simulink model "gate_V31_2016a"  (R2016a) (continued)
== system gate_V31_2016a/Gate/HardwareInputWrapper/MIL_Gate_A == (continued)
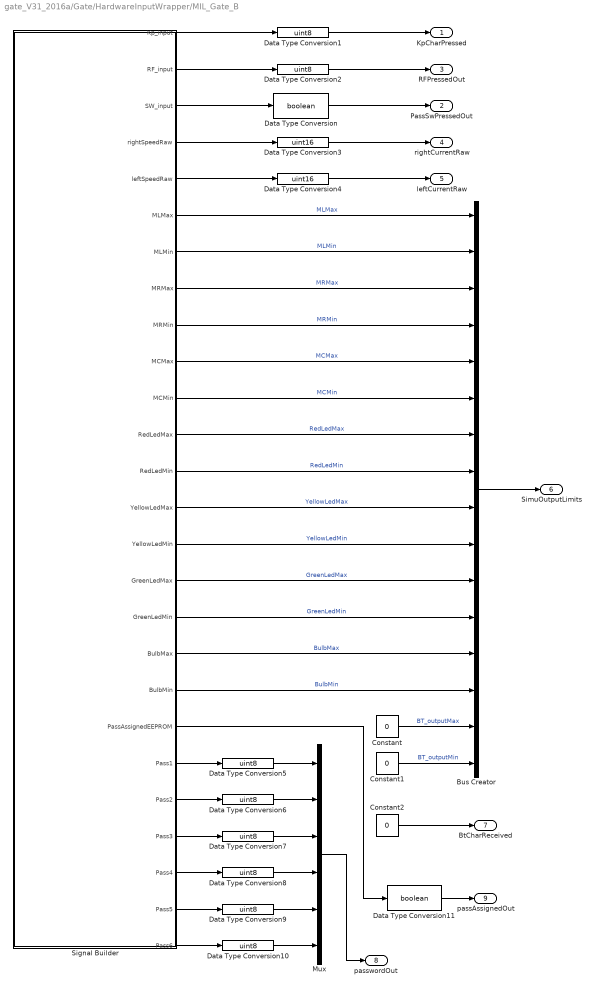
== system gate_V31_2016a/Gate/HardwareInputWrapper/MIL_Gate_B ==
  Bus Creator : BusCreator  Inputs=16
  Constant : Constant  Value=0
  Constant1 : Constant  Value=0
  Constant2 : Constant  Value=0
  Data Type Conversion : DataTypeConversion  OutDataTypeStr=boolean
  Data Type Conversion{1..2,5..10} (8 alike) : DataTypeConversion  OutDataTypeStr=uint8
  Data Type Conversion11 : DataTypeConversion  OutDataTypeStr=boolean
  Data Type Conversion3 : DataTypeConversion  OutDataTypeStr=uint16
  Data Type Conversion4 : DataTypeConversion  OutDataTypeStr=uint16
  Mux : Mux  Inputs=6
  Signal Builder : Subsystem  Tag=STV Subsys  LoadFcn=sigbuilder_block('load',[6 6 1012.5 507 ]);  StartFcn=sigbuilder_block('start');  StopFcn=sigbuilder_block('stop');  ClipboardFcn=sigbuilder_block('clipboard');  mask(Sigbuilder block){}
      mask help: Simulink:masks:SigbuilderBlock_MD
      mask init:
      | if ~strcmp(get_param(bdroot(gcbh),'SimulationStatus'),'stopped') tuvar = sigbuilder_block('maskInit'); end
      settings: CopyFcn=sigbuilder_block('copy');  DeleteFcn=sigbuilder_block('delete');  ModelCloseFcn=sigbuilder_block('modelClose');  PreSaveFcn=sigbuilder_block('preSave');  PauseFcn=sigbuilder_block('pause');  ContinueFcn=sigbuilder_block('continue');  NameChangeFcn=sigbuilder_block('namechange');  OpenFcn=sigbuilder_block('open',[-1152 0 1152 592.8 ]);  CloseFcn=sigbuilder_block('close');
      mask Display: plot(0, 0, 100, 100,[2, 2, 32, 32, 2], [68, 8, 8, 68, 68],[32, 2], [38, 38], [32, 19, 2],[53, 60, 44], [32, 17, 17, 2],[16, 16, 31, 31]);txt = getActiveGroup(gcbh);text(2, 100, txt,'verticalAlignment', 'top');
  KpCharPressed : Outport
  PassSwPressedOut : Outport  Port=2
  RFPressedOut : Outport  Port=3
  rightCurrentRaw : Outport  Port=4
  leftCurrentRaw : Outport  Port=5
  SimuOutputLimits : Outport  Port=6
  BtCharReceived : Outport  Port=7
  passwordOut : Outport  Port=8
  passAssignedOut : Outport  Port=9
  wires:
  Signal Builder/5(leftSpeedRaw) -> Data Type Conversion4/1
  Signal Builder/4(rightSpeedRaw) -> Data Type Conversion3/1
  Signal Builder/6(MLMax) -> Bus Creator/1  "MLMax"
  Signal Builder/7(MLMin) -> Bus Creator/2  "MLMin"
  Signal Builder/8(MRMax) -> Bus Creator/3  "MRMax"
  Signal Builder/9(MRMin) -> Bus Creator/4  "MRMin"
  Signal Builder/10(MCMax) -> Bus Creator/5  "MCMax"
  Signal Builder/11(MCMin) -> Bus Creator/6  "MCMin"
  Signal Builder/12(RedLedMax) -> Bus Creator/7  "RedLedMax"
  Signal Builder/19(BulbMin) -> Bus Creator/14  "BulbMin"
  Signal Builder/13(RedLedMin) -> Bus Creator/8  "RedLedMin"
  Signal Builder/18(BulbMax) -> Bus Creator/13  "BulbMax"
  Signal Builder/14(YellowLedMax) -> Bus Creator/9  "YellowLedMax"
  Signal Builder/17(GreenLedMin) -> Bus Creator/12  "GreenLedMin"
  Signal Builder/15(YellowLedMin) -> Bus Creator/10  "YellowLedMin"
  Signal Builder/16(GreenLedMax) -> Bus Creator/11  "GreenLedMax"
  Bus Creator/1 -> SimuOutputLimits/1
  Signal Builder/3(SW_input) -> Data Type Conversion/1
  Signal Builder/2(RF_input) -> Data Type Conversion2/1
  Signal Builder/1(Kp_input) -> Data Type Conversion1/1
  Constant/1 -> Bus Creator/15  "BT_outputMax"
  Constant1/1 -> Bus Creator/16  "BT_outputMin"
  Constant2/1 -> BtCharReceived/1
  Data Type Conversion/1 -> PassSwPressedOut/1
  Signal Builder/20(PassAssignedEEPROM) -> Data Type Conversion11/1
  Signal Builder/23(Pass3) -> Data Type Conversion7/1
  Signal Builder/22(Pass2) -> Data Type Conversion6/1
  Signal Builder/25(Pass5) -> Data Type Conversion9/1
  Signal Builder/21(Pass1) -> Data Type Conversion5/1
  Signal Builder/26(Pass6) -> Data Type Conversion10/1
  Signal Builder/24(Pass4) -> Data Type Conversion8/1
  Mux/1 -> passwordOut/1
  Data Type Conversion1/1 -> KpCharPressed/1
  Data Type Conversion2/1 -> RFPressedOut/1
  Data Type Conversion3/1 -> rightCurrentRaw/1
  Data Type Conversion4/1 -> leftCurrentRaw/1
  Data Type Conversion5/1 -> Mux/1
  Data Type Conversion6/1 -> Mux/2
  Data Type Conversion7/1 -> Mux/3
  Data Type Conversion8/1 -> Mux/4
  Data Type Conversion9/1 -> Mux/5
  Data Type Conversion10/1 -> Mux/6
  Data Type Conversion11/1 -> passAssignedOut/1

== system gate_V31_2016a/Gate/HardwareInputWrapper/MIL_Gate_C ==
  Bus Creator : BusCreator  Inputs=16
  Constant{,1..9} (10 alike) : Constant  Value=0
  Signal Builder : Subsystem  Tag=STV Subsys  LoadFcn=sigbuilder_block('load');  StartFcn=sigbuilder_block('start');  StopFcn=sigbuilder_block('stop');  ClipboardFcn=sigbuilder_block('clipboard');  mask(Sigbuilder block){}
      mask help: Simulink:masks:SigbuilderBlock_MD
      mask init:
      | if ~strcmp(get_param(bdroot(gcbh),'SimulationStatus'),'stopped') tuvar = sigbuilder_block('maskInit'); end
      settings: CopyFcn=sigbuilder_block('copy');  DeleteFcn=sigbuilder_block('delete');  ModelCloseFcn=sigbuilder_block('modelClose');  PreSaveFcn=sigbuilder_block('preSave');  PauseFcn=sigbuilder_block('pause');  ContinueFcn=sigbuilder_block('continue');  NameChangeFcn=sigbuilder_block('namechange');  OpenFcn=sigbuilder_block('open',[6 6 1012.5 507 ]);  CloseFcn=sigbuilder_block('close');
      mask Display: plot(0, 0, 100, 100,[2, 2, 32, 32, 2], [68, 8, 8, 68, 68],[32, 2], [38, 38], [32, 19, 2],[53, 60, 44], [32, 17, 17, 2],[16, 16, 31, 31]);txt = getActiveGroup(gcbh);text(2, 100, txt,'verticalAlignment', 'top');
  KpCharPressed : Outport
  BtCharReceived : Outport  Port=2
  PassSwPressedOut : Outport  Port=3
  RFPressedOut : Outport  Port=4
  rightCurrentRaw : Outport  Port=5
  leftCurrentRaw : Outport  Port=6
  SimuOutputLimits : Outport  Port=7
  wires:
  Constant8/1 -> KpCharPressed/1
  Constant9/1 -> BtCharReceived/1
  Signal Builder/1(RF_input) -> RFPressedOut/1
  Signal Builder/2(SW_input) -> PassSwPressedOut/1
  Bus Creator/1 -> SimuOutputLimits/1
  Constant4/1 -> Bus Creator/11  "GreenLedMax"
  Constant3/1 -> Bus Creator/10  "YellowLedMin"
  Constant5/1 -> Bus Creator/12  "GreenLedMin"
  Constant2/1 -> Bus Creator/9  "YellowLedMax"
  Signal Builder/11(BulbMax) -> Bus Creator/13  "BulbMax"
  Constant1/1 -> Bus Creator/8  "RedLedMin"
  Signal Builder/12(BulbMin) -> Bus Creator/14  "BulbMin"
  Constant6/1 -> Bus Creator/15  "BT_outputMax"
  Constant/1 -> Bus Creator/7  "RedLedMax"
  Signal Builder/10(MCMin) -> Bus Creator/6  "MCMin"
  Signal Builder/9(MCMax) -> Bus Creator/5  "MCMax"
  Signal Builder/8(MRMin) -> Bus Creator/4  "MRMin"
  Signal Builder/7(MRMax) -> Bus Creator/3  "MRMax"
  Signal Builder/6(MLMin) -> Bus Creator/2  "MLMin"
  Signal Builder/5(MLMax) -> Bus Creator/1  "MLMax"
  Constant7/1 -> Bus Creator/16  "BT_outputMin"
  Signal Builder/3(rightSpeedRaw) -> rightCurrentRaw/1
  Signal Builder/4(leftSpeedRaw) -> leftCurrentRaw/1

== system gate_V31_2016a/Gate/outputPinsBlock/HIL ==
  greenLedPin : Inport
  redLedPin : Inport  Port=2
  yellowLed : Inport  Port=3
  leftMotorPin : Inport  Port=4
  commonMotorPin : Inport  Port=5
  rightMotorPin : Inport  Port=6
  bulbPin : Inport  Port=7
  Digital Output : arduinolib/Digital Output  pinNumber=11  blockPlatform=arduino
  Digital Output1 : arduinolib/Digital Output  pinNumber=12  blockPlatform=arduino
  Digital Output2 : arduinolib/Digital Output  pinNumber=13  blockPlatform=arduino
  S-Function Builder1 : S-Function  InitFcn=try, set_param(gcb,'FunctionName','A2PIN_Lib'), end  FunctionName=A2PIN_Lib  SFunctionModules=A2PIN_Lib_wrapper  SFunctionDeploymentMode=off  EnableBusSupport=off  mask(S-Function Builder){}
      mask help: Simulink:masks:SFunctionBuilder_MD
      mask init: same as gate_V31_2016a/Gate/HardwareInputWrapper/HIL/S-Function Builder1
      settings: CopyFcn=set_param(gcbh,'LinkStatus','none')  PreSaveFcn=try, set_param(gcb,'FunctionName','A2PIN_Lib'), end  OpenFcn=sfunctionwizard(gcbh)
      mask Display: plot(val(:,1),val(:,2)),disp(sys), port_label('input',1,'input')
  S-Function Builder2 : S-Function  InitFcn=try, set_param(gcb,'FunctionName','A3PIN_Lib'), end  FunctionName=A3PIN_Lib  SFunctionModules=A3PIN_Lib_wrapper  SFunctionDeploymentMode=off  EnableBusSupport=off  mask(S-Function Builder){}
      mask help: Simulink:masks:SFunctionBuilder_MD
      mask init: same as gate_V31_2016a/Gate/HardwareInputWrapper/HIL/S-Function Builder1
      settings: CopyFcn=set_param(gcbh,'LinkStatus','none')  PreSaveFcn=try, set_param(gcb,'FunctionName','A3PIN_Lib'), end  OpenFcn=sfunctionwizard(gcbh)
      mask Display: plot(val(:,1),val(:,2)),disp(sys), port_label('input',1,'input')
  S-Function Builder3 : S-Function  InitFcn=try, set_param(gcb,'FunctionName','A4PIN_Lib'), end  FunctionName=A4PIN_Lib  SFunctionModules=A4PIN_Lib_wrapper  SFunctionDeploymentMode=off  EnableBusSupport=off  mask(S-Function Builder){}
      mask help: Simulink:masks:SFunctionBuilder_MD
      mask init: same as gate_V31_2016a/Gate/HardwareInputWrapper/HIL/S-Function Builder1
      settings: CopyFcn=set_param(gcbh,'LinkStatus','none')  PreSaveFcn=try, set_param(gcb,'FunctionName','A4PIN_Lib'), end  OpenFcn=sfunctionwizard(gcbh)
      mask Display: plot(val(:,1),val(:,2)),disp(sys), port_label('input',1,'input')
  S-Function Builder4 : S-Function  InitFcn=try, set_param(gcb,'FunctionName','A5PIN_Lib'), end  FunctionName=A5PIN_Lib  SFunctionModules=A5PIN_Lib_wrapper  SFunctionDeploymentMode=off  EnableBusSupport=off  mask(S-Function Builder){}
      mask help: Simulink:masks:SFunctionBuilder_MD
      mask init: same as gate_V31_2016a/Gate/HardwareInputWrapper/HIL/S-Function Builder1
      settings: CopyFcn=set_param(gcbh,'LinkStatus','none')  PreSaveFcn=try, set_param(gcb,'FunctionName','A5PIN_Lib'), end  OpenFcn=sfunctionwizard(gcbh)
      mask Display: plot(val(:,1),val(:,2)),disp(sys), port_label('input',1,'input')
  wires:
  yellowLed/1 -> Digital Output2/1
  redLedPin/1 -> Digital Output/1
  greenLedPin/1 -> Digital Output1/1
  commonMotorPin/1 -> S-Function Builder3/1
  leftMotorPin/1 -> S-Function Builder2/1
  rightMotorPin/1 -> S-Function Builder4/1
  bulbPin/1 -> S-Function Builder1/1

== system gate_V31_2016a/Gate/outputPinsBlock/MIL ==
  "leftMotorPin " : Inport
  "commonMotorPin " : Inport  Port=2
  "rightMotorPin " : Inport  Port=3
  greenLedPin : Inport  Port=4
  redLedPin : Inport  Port=5
  yellowLed : Inport  Port=6
  bulbPin : Inport  Port=7
  BT_TxChar : Inport  Port=8
  SimuOutputLimits : Inport  Port=9
  ScopeBlocks : Subsystem  VariantControl=VisualOutputs
  "VerificationBlocks " : Subsystem  VariantControl=VVOutputs

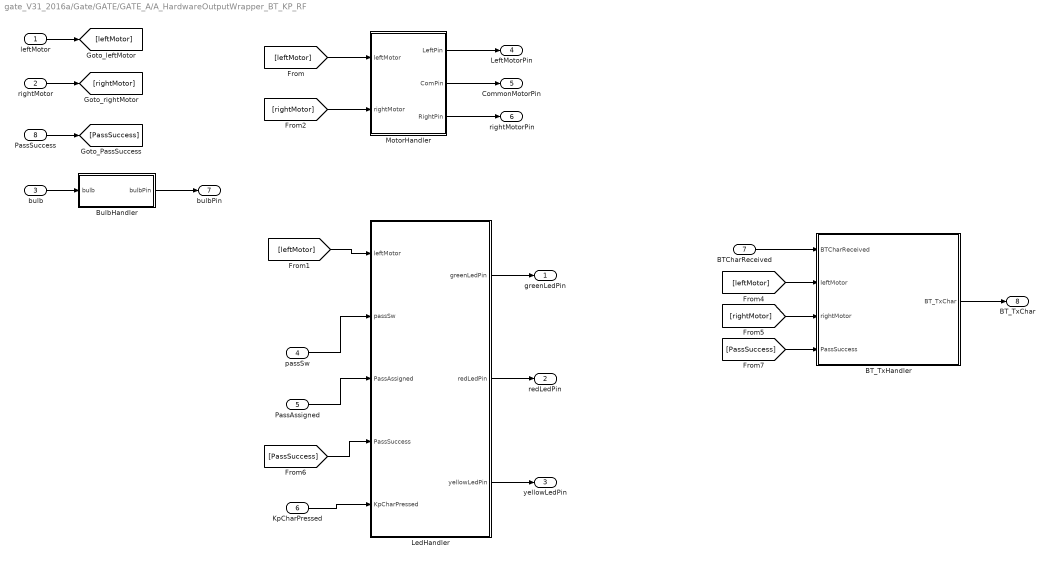
== system gate_V31_2016a/Gate/GATE/GATE_A/A_HardwareOutputWrapper_BT_KP_RF ==
  leftMotor : Inport
  rightMotor : Inport  Port=2
  bulb : Inport  Port=3
  passSw : Inport  Port=4
  PassAssigned : Inport  Port=5
  KpCharPressed : Inport  Port=6
  BTCharReceived : Inport  Port=7
  PassSuccess : Inport  Port=8
  BT_TxHandler : Subsystem
  BulbHandler : Subsystem
  LedHandler : Subsystem
  MotorHandler : Subsystem
  greenLedPin : Outport
  redLedPin : Outport  Port=2
  yellowLedPin : Outport  Port=3
  LeftMotorPin : Outport  Port=4
  CommonMotorPin : Outport  Port=5
  rightMotorPin : Outport  Port=6
  bulbPin : Outport  Port=7
  BT_TxChar : Outport  Port=8
  wires:
  [leftMotor] -> MotorHandler/1(leftMotor)
  [rightMotor] -> MotorHandler/2(rightMotor)
  bulb/1 -> BulbHandler/1(bulb)
  LedHandler/1(greenLedPin) -> greenLedPin/1
  LedHandler/2(redLedPin) -> redLedPin/1
  LedHandler/3(yellowLedPin) -> yellowLedPin/1
  MotorHandler/1(LeftPin) -> LeftMotorPin/1
  MotorHandler/2(ComPin) -> CommonMotorPin/1
  MotorHandler/3(RightPin) -> rightMotorPin/1
  BulbHandler/1(bulbPin) -> bulbPin/1
  leftMotor/1 -> [leftMotor]
  [leftMotor] -> LedHandler/1(leftMotor)
  rightMotor/1 -> [rightMotor]
  passSw/1 -> LedHandler/2(passSw)
  PassAssigned/1 -> LedHandler/3(PassAssigned)
  [PassSuccess] -> LedHandler/4(PassSuccess)
  KpCharPressed/1 -> LedHandler/5(KpCharPressed)
  [leftMotor] -> BT_TxHandler/2(leftMotor)
  BTCharReceived/1 -> BT_TxHandler/1(BTCharReceived)
  [rightMotor] -> BT_TxHandler/3(rightMotor)
  PassSuccess/1 -> [PassSuccess]
  [PassSuccess] -> BT_TxHandler/4(PassSuccess)
  BT_TxHandler/1(BT_TxChar) -> BT_TxChar/1

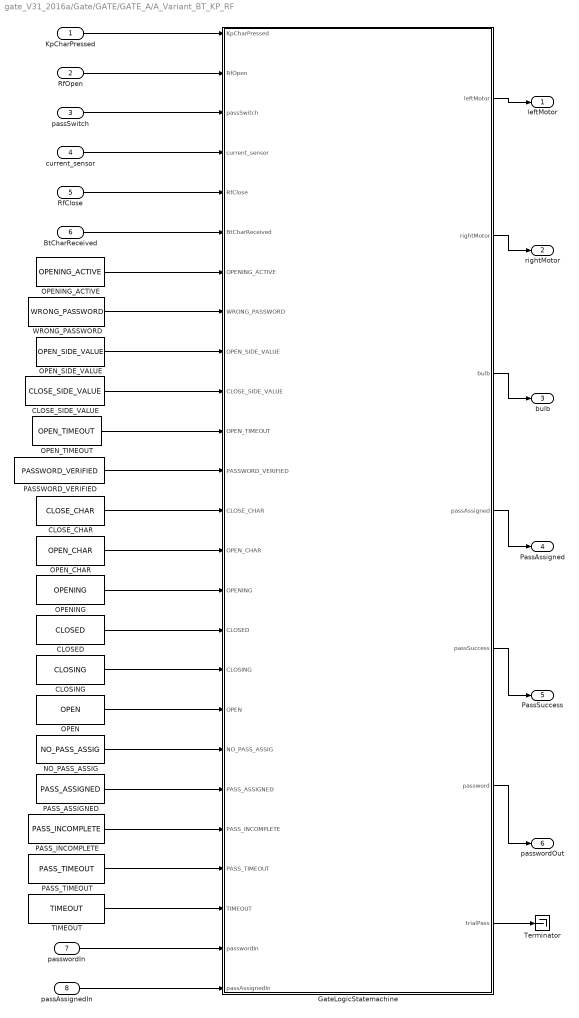
== system gate_V31_2016a/Gate/GATE/GATE_A/A_Variant_BT_KP_RF ==
  KpCharPressed : Inport
  RfOpen : Inport  Port=2
  passSwitch : Inport  Port=3
  current_sensor : Inport  Port=4
  RfClose : Inport  Port=5
  BtCharReceived : Inport  Port=6
  passwordIn : Inport  Port=7
  passAssignedIn : Inport  Port=8
  CLOSED : Constant  Value=CLOSED  SampleTime=-1
  CLOSE_CHAR : Constant  Value=CLOSE_CHAR  SampleTime=-1
  CLOSE_SIDE_VALUE : Constant  Value=CLOSE_SIDE_VALUE  SampleTime=-1
  CLOSING : Constant  Value=CLOSING  SampleTime=-1
  GateLogicStatemachine : Stateflow chart  TreatAsAtomicUnit=on
  NO_PASS_ASSIG : Constant  Value=NO_PASS_ASSIG  SampleTime=-1
  OPEN : Constant  Value=OPEN  SampleTime=-1
  OPENING : Constant  Value=OPENING  SampleTime=-1
  OPENING_ACTIVE : Constant  Value=OPENING_ACTIVE  SampleTime=-1
  OPEN_CHAR : Constant  Value=OPEN_CHAR  SampleTime=-1
  OPEN_SIDE_VALUE : Constant  Value=OPEN_SIDE_VALUE  SampleTime=-1
  OPEN_TIMEOUT : Constant  Value=OPEN_TIMEOUT  SampleTime=-1
  PASSWORD_VERIFIED : Constant  Value=PASSWORD_VERIFIED  SampleTime=-1
  PASS_ASSIGNED : Constant  Value=PASS_ASSIGNED  SampleTime=-1
  PASS_INCOMPLETE : Constant  Value=PASS_INCOMPLETE  SampleTime=-1
  PASS_TIMEOUT : Constant  Value=PASS_TIMEOUT  SampleTime=-1
  TIMEOUT : Constant  Value=TIMEOUT  SampleTime=-1
  Terminator : Terminator
  WRONG_PASSWORD : Constant  Value=WRONG_PASSWORD  SampleTime=-1
  leftMotor : Outport
  rightMotor : Outport  Port=2
  bulb : Outport  Port=3
  PassAssigned : Outport  Port=4
  PassSuccess : Outport  Port=5
  passwordOut : Outport  Port=6
  wires:
  RfClose/1 -> GateLogicStatemachine/5(RfClose)
  TIMEOUT/1 -> GateLogicStatemachine/23(TIMEOUT)
  PASS_INCOMPLETE/1 -> GateLogicStatemachine/21(PASS_INCOMPLETE)
  PASS_ASSIGNED/1 -> GateLogicStatemachine/20(PASS_ASSIGNED)
  GateLogicStatemachine/4(passAssigned) -> PassAssigned/1
  passSwitch/1 -> GateLogicStatemachine/3(passSwitch)
  GateLogicStatemachine/5(passSuccess) -> PassSuccess/1
  PASS_TIMEOUT/1 -> GateLogicStatemachine/22(PASS_TIMEOUT)
  KpCharPressed/1 -> GateLogicStatemachine/1(KpCharPressed)
  BtCharReceived/1 -> GateLogicStatemachine/6(BtCharReceived)
  NO_PASS_ASSIG/1 -> GateLogicStatemachine/19(NO_PASS_ASSIG)
  CLOSE_SIDE_VALUE/1 -> GateLogicStatemachine/10(CLOSE_SIDE_VALUE)
  OPEN_TIMEOUT/1 -> GateLogicStatemachine/11(OPEN_TIMEOUT)
  OPEN_SIDE_VALUE/1 -> GateLogicStatemachine/9(OPEN_SIDE_VALUE)
  OPENING_ACTIVE/1 -> GateLogicStatemachine/7(OPENING_ACTIVE)
  GateLogicStatemachine/2(rightMotor) -> rightMotor/1
  GateLogicStatemachine/1(leftMotor) -> leftMotor/1
  WRONG_PASSWORD/1 -> GateLogicStatemachine/8(WRONG_PASSWORD)
  current_sensor/1 -> GateLogicStatemachine/4(current_sensor)
  GateLogicStatemachine/3(bulb) -> bulb/1
  RfOpen/1 -> GateLogicStatemachine/2(RfOpen)
  PASSWORD_VERIFIED/1 -> GateLogicStatemachine/12(PASSWORD_VERIFIED)
  OPEN/1 -> GateLogicStatemachine/18(OPEN)
  CLOSE_CHAR/1 -> GateLogicStatemachine/13(CLOSE_CHAR)
  OPEN_CHAR/1 -> GateLogicStatemachine/14(OPEN_CHAR)
  CLOSED/1 -> GateLogicStatemachine/16(CLOSED)
  OPENING/1 -> GateLogicStatemachine/15(OPENING)
  CLOSING/1 -> GateLogicStatemachine/17(CLOSING)
  GateLogicStatemachine/6(password) -> passwordOut/1
  passwordIn/1 -> GateLogicStatemachine/24(passwordIn)
  passAssignedIn/1 -> GateLogicStatemachine/25(passAssignedIn)
  GateLogicStatemachine/7(trialPass) -> Terminator/1

== system gate_V31_2016a/Gate/GATE/GATE_A/CurrentFilterSubsystem ==
  rightCurrentRaw : Inport
  leftCurrentRaw : Inport  Port=2
  10msSmapler : Stateflow chart  TreatAsAtomicUnit=on
  Subsystem : Subsystem
  current : Outport
  wires:
  10msSmapler/1(triggerOn()) -> Subsystem/Trigger
  Subsystem/1(current) -> current/1
  rightCurrentRaw/1 -> Subsystem/1(rightCurrentRaw)
  leftCurrentRaw/1 -> Subsystem/2(leftCurrentRaw)

== system gate_V31_2016a/Gate/GATE/GATE_A/RFHandler ==
  RFInput : Inport
  leftMotor : Inport  Port=2
  rightMotor : Inport  Port=3
  CLOSED : Constant  Value=CLOSED  SampleTime=-1
  CLOSING : Constant  Value=CLOSING  SampleTime=-1
  OPEN : Constant  Value=OPEN  SampleTime=-1
  OPENING : Constant  Value=OPENING  SampleTime=-1
  RFHandlerL : Stateflow chart  TreatAsAtomicUnit=on
  RFOpen : Outport
  RFClose : Outport  Port=2
  wires:
  RFInput/1 -> RFHandlerL/1(btn)
  RFHandlerL/1(RFClose) -> RFClose/1
  RFHandlerL/2(RFOpen) -> RFOpen/1
  OPEN/1 -> RFHandlerL/6(OPEN)
  CLOSING/1 -> RFHandlerL/5(CLOSING)
  CLOSED/1 -> RFHandlerL/4(CLOSED)
  OPENING/1 -> RFHandlerL/7(OPENING)
  leftMotor/1 -> RFHandlerL/2(leftMotor)
  rightMotor/1 -> RFHandlerL/3(rightMotor)

== system gate_V31_2016a/Gate/GATE/GATE_B/B_HardwareOutputWrapper_KP_RF ==
  leftMotor : Inport
  rightMotor : Inport  Port=2
  bulbPinIn_BOOL : Inport  Port=3
  passSw : Inport  Port=4
  PassAssigned : Inport  Port=5
  KpCharPressed : Inport  Port=6
  BTCharReceived : Inport  Port=7
  PassSuccess : Inport  Port=8
  Constant : Constant  Value=0  SampleTime=-1
  LedHandler : Subsystem
  MotorHandler : Subsystem
  Terminator : Terminator
  greenLedPin : Outport
  redLedPin : Outport  Port=2
  yellowLedPin : Outport  Port=3
  LeftMotorPin : Outport  Port=4
  CommonMotorPin : Outport  Port=5
  rightMotorPin : Outport  Port=6
  bulbPin_BOOL : Outport  Port=7
  BT_TxChar : Outport  Port=8
  wires:
  rightMotor/1 -> MotorHandler/2(rightMotor)
  BTCharReceived/1 -> Terminator/1
  [leftMotor] -> MotorHandler/1(leftMotor)
  LedHandler/1(greenLedPin) -> greenLedPin/1
  LedHandler/2(redLedPin) -> redLedPin/1
  LedHandler/3(yellowLedPin) -> yellowLedPin/1
  MotorHandler/1(LeftPin) -> LeftMotorPin/1
  MotorHandler/2(ComPin) -> CommonMotorPin/1
  MotorHandler/3(RightPin) -> rightMotorPin/1
  bulbPinIn_BOOL/1 -> bulbPin_BOOL/1
  leftMotor/1 -> [leftMotor]
  [leftMotor] -> LedHandler/1(leftMotor)
  passSw/1 -> LedHandler/2(passSw)
  PassAssigned/1 -> LedHandler/3(PassAssigned)
  [PassSuccess] -> LedHandler/4(PassSuccess)
  KpCharPressed/1 -> LedHandler/5(KpCharPressed)
  PassSuccess/1 -> [PassSuccess]
  Constant/1 -> BT_TxChar/1

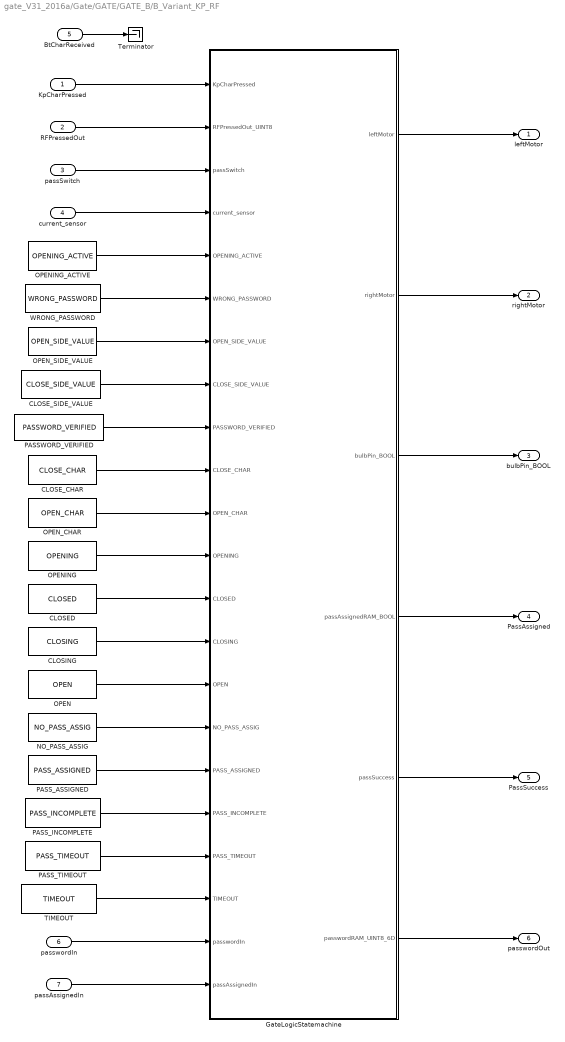
== system gate_V31_2016a/Gate/GATE/GATE_B/B_Variant_KP_RF ==
  KpCharPressed : Inport
  RFPressedOut : Inport  Port=2
  passSwitch : Inport  Port=3
  current_sensor : Inport  Port=4
  BtCharReceived : Inport  Port=5
  passwordIn : Inport  Port=6
  passAssignedIn : Inport  Port=7
  CLOSED : Constant  Value=CLOSED  SampleTime=-1
  CLOSE_CHAR : Constant  Value=CLOSE_CHAR  SampleTime=-1
  CLOSE_SIDE_VALUE : Constant  Value=CLOSE_SIDE_VALUE  SampleTime=-1
  CLOSING : Constant  Value=CLOSING  SampleTime=-1
  GateLogicStatemachine : Stateflow chart  TreatAsAtomicUnit=on
  NO_PASS_ASSIG : Constant  Value=NO_PASS_ASSIG  SampleTime=-1
  OPEN : Constant  Value=OPEN  SampleTime=-1
  OPENING : Constant  Value=OPENING  SampleTime=-1
  OPENING_ACTIVE : Constant  Value=OPENING_ACTIVE  SampleTime=-1
  OPEN_CHAR : Constant  Value=OPEN_CHAR  SampleTime=-1
  OPEN_SIDE_VALUE : Constant  Value=OPEN_SIDE_VALUE  SampleTime=-1
  PASSWORD_VERIFIED : Constant  Value=PASSWORD_VERIFIED  SampleTime=-1
  PASS_ASSIGNED : Constant  Value=PASS_ASSIGNED  SampleTime=-1
  PASS_INCOMPLETE : Constant  Value=PASS_INCOMPLETE  SampleTime=-1
  PASS_TIMEOUT : Constant  Value=PASS_TIMEOUT  SampleTime=-1
  TIMEOUT : Constant  Value=TIMEOUT  SampleTime=-1
  Terminator : Terminator
  WRONG_PASSWORD : Constant  Value=WRONG_PASSWORD  SampleTime=-1
  leftMotor : Outport
  rightMotor : Outport  Port=2
  bulbPin_BOOL : Outport  Port=3
  PassAssigned : Outport  Port=4
  PassSuccess : Outport  Port=5
  passwordOut : Outport  Port=6
  wires:
  GateLogicStatemachine/6(passwordRAM_UINT8_6D) -> passwordOut/1
  passAssignedIn/1 -> GateLogicStatemachine/22(passAssignedIn)
  passwordIn/1 -> GateLogicStatemachine/21(passwordIn)
  BtCharReceived/1 -> Terminator/1
  TIMEOUT/1 -> GateLogicStatemachine/20(TIMEOUT)
  PASS_INCOMPLETE/1 -> GateLogicStatemachine/18(PASS_INCOMPLETE)
  PASS_ASSIGNED/1 -> GateLogicStatemachine/17(PASS_ASSIGNED)
  GateLogicStatemachine/4(passAssignedRAM_BOOL) -> PassAssigned/1
  passSwitch/1 -> GateLogicStatemachine/3(passSwitch)
  GateLogicStatemachine/5(passSuccess) -> PassSuccess/1
  PASS_TIMEOUT/1 -> GateLogicStatemachine/19(PASS_TIMEOUT)
  KpCharPressed/1 -> GateLogicStatemachine/1(KpCharPressed)
  NO_PASS_ASSIG/1 -> GateLogicStatemachine/16(NO_PASS_ASSIG)
  CLOSE_SIDE_VALUE/1 -> GateLogicStatemachine/8(CLOSE_SIDE_VALUE)
  OPEN_SIDE_VALUE/1 -> GateLogicStatemachine/7(OPEN_SIDE_VALUE)
  OPENING_ACTIVE/1 -> GateLogicStatemachine/5(OPENING_ACTIVE)
  GateLogicStatemachine/2(rightMotor) -> rightMotor/1
  GateLogicStatemachine/1(leftMotor) -> leftMotor/1
  WRONG_PASSWORD/1 -> GateLogicStatemachine/6(WRONG_PASSWORD)
  current_sensor/1 -> GateLogicStatemachine/4(current_sensor)
  GateLogicStatemachine/3(bulbPin_BOOL) -> bulbPin_BOOL/1
  RFPressedOut/1 -> GateLogicStatemachine/2(RFPressedOut_UINT8)
  PASSWORD_VERIFIED/1 -> GateLogicStatemachine/9(PASSWORD_VERIFIED)
  OPEN/1 -> GateLogicStatemachine/15(OPEN)
  CLOSE_CHAR/1 -> GateLogicStatemachine/10(CLOSE_CHAR)
  OPEN_CHAR/1 -> GateLogicStatemachine/11(OPEN_CHAR)
  CLOSED/1 -> GateLogicStatemachine/13(CLOSED)
  OPENING/1 -> GateLogicStatemachine/12(OPENING)
  CLOSING/1 -> GateLogicStatemachine/14(CLOSING)

== system gate_V31_2016a/Gate/GATE/GATE_B/CurrentFilterSubsystem ==
  rightCurrentRaw : Inport
  leftCurrentRaw : Inport  Port=2
  10msSmapler : Stateflow chart  Commented=on  TreatAsAtomicUnit=on
  Subsystem : Subsystem
  current : Outport
  rightCurr : Outport  Port=2
  leftCurr : Outport  Port=3
  wires:
  Subsystem/1(current) -> current/1
  rightCurrentRaw/1 -> Subsystem/1(rightCurrentRaw)
  leftCurrentRaw/1 -> Subsystem/2(leftCurrentRaw)
  Subsystem/2(rightCurr) -> rightCurr/1
  Subsystem/3(leftCurr) -> leftCurr/1

== system gate_V31_2016a/Gate/GATE/GATE_B/Subsystem1 ==
  In1 : Inport
  In2 : Inport  Port=2
  In3 : Inport  Port=3
  In4 : Inport  Port=4
  In5 : Inport  Port=5
  In6 : Inport  Port=6
  10msSmapler : Stateflow chart  TreatAsAtomicUnit=on
  Subsystem : Subsystem
  wires:
  In3/1 -> Subsystem/3(In3)
  In1/1 -> Subsystem/1(In1)
  In2/1 -> Subsystem/2(In2)
  In5/1 -> Subsystem/5(In5)
  In6/1 -> Subsystem/6(In6)
  10msSmapler/1(triggerOn()) -> Subsystem/Trigger
  In4/1 -> Subsystem/4(In4)

== system gate_V31_2016a/Gate/GATE/GATE_C/C_HardwareOutputWrapper_RF ==
  leftMotor : Inport
  rightMotor : Inport  Port=2
  bulbPinIn_BOOL : Inport  Port=3
  passSw : Inport  Port=4
  Constant{,1..3} (4 alike) : Constant  Value=0  SampleTime=-1
  MotorHandler : Subsystem
  Terminator1 : Terminator
  greenLedPin : Outport
  redLedPin : Outport  Port=2
  yellowLedPin : Outport  Port=3
  LeftMotorPin : Outport  Port=4
  CommonMotorPin : Outport  Port=5
  rightMotorPin : Outport  Port=6
  bulbPin_BOOL : Outport  Port=7
  BT_TxChar : Outport  Port=8
  wires:
  [leftMotor] -> MotorHandler/1(leftMotor)
  [rightMotor] -> MotorHandler/2(rightMotor)
  MotorHandler/1(LeftPin) -> LeftMotorPin/1
  MotorHandler/2(ComPin) -> CommonMotorPin/1
  MotorHandler/3(RightPin) -> rightMotorPin/1
  bulbPinIn_BOOL/1 -> bulbPin_BOOL/1
  leftMotor/1 -> [leftMotor]
  rightMotor/1 -> [rightMotor]
  Constant/1 -> BT_TxChar/1
  Constant3/1 -> yellowLedPin/1
  Constant2/1 -> redLedPin/1
  Constant1/1 -> greenLedPin/1
  passSw/1 -> Terminator1/1

== system gate_V31_2016a/Gate/GATE/GATE_C/C_Variant_RF ==
  RFPressedOut_UINT8 : Inport
  passSwitch : Inport  Port=2
  current_sensor : Inport  Port=3
  CLOSED : Constant  Value=CLOSED  SampleTime=-1
  CLOSE_SIDE_VALUE : Constant  Value=CLOSE_SIDE_VALUE  SampleTime=-1
  CLOSING : Constant  Value=CLOSING  SampleTime=-1
  GateLogicStatemachine : Stateflow chart  TreatAsAtomicUnit=on
  OPEN : Constant  Value=OPEN  SampleTime=-1
  OPENING : Constant  Value=OPENING  SampleTime=-1
  OPENING_ACTIVE : Constant  Value=OPENING_ACTIVE  SampleTime=-1
  OPEN_SIDE_VALUE : Constant  Value=OPEN_SIDE_VALUE  SampleTime=-1
  leftMotor : Outport
  rightMotor : Outport  Port=2
  bulbPin_BOOL : Outport  Port=3
  wires:
  passSwitch/1 -> GateLogicStatemachine/2(passSwitch)
  CLOSE_SIDE_VALUE/1 -> GateLogicStatemachine/6(CLOSE_SIDE_VALUE)
  OPEN_SIDE_VALUE/1 -> GateLogicStatemachine/5(OPEN_SIDE_VALUE)
  OPENING_ACTIVE/1 -> GateLogicStatemachine/4(OPENING_ACTIVE)
  GateLogicStatemachine/2(rightMotor) -> rightMotor/1
  GateLogicStatemachine/1(leftMotor) -> leftMotor/1
  current_sensor/1 -> GateLogicStatemachine/3(current_sensor)
  GateLogicStatemachine/3(bulbPin_BOOL) -> bulbPin_BOOL/1
  RFPressedOut_UINT8/1 -> GateLogicStatemachine/1(RFPressedOut_UINT8)
  OPEN/1 -> GateLogicStatemachine/10(OPEN)
  CLOSED/1 -> GateLogicStatemachine/8(CLOSED)
  OPENING/1 -> GateLogicStatemachine/7(OPENING)
  CLOSING/1 -> GateLogicStatemachine/9(CLOSING)

== system gate_V31_2016a/Gate/GATE/GATE_C/CurrentFilterSubsystem ==
  rightCurrentRaw : Inport
  leftCurrentRaw : Inport  Port=2
  10msSmapler : Stateflow chart  TreatAsAtomicUnit=on
  Subsystem : Subsystem
  current : Outport
  wires:
  rightCurrentRaw/1 -> Subsystem/1(rightCurrentRaw)
  Subsystem/1(current) -> current/1
  10msSmapler/1(triggerOn()) -> Subsystem/Trigger
  leftCurrentRaw/1 -> Subsystem/2(leftCurrentRaw)

== system gate_V31_2016a/Gate/HardwareInputWrapper/HIL/BluetoothWrapper ==
  S-Function Builder : S-Function  InitFcn=try, set_param(gcb,'FunctionName','BluetoothLib'), end  FunctionName=BluetoothLib  SFunctionModules=BluetoothLib_wrapper  SFunctionDeploymentMode=off  EnableBusSupport=off  mask(S-Function Builder){}
      mask help: Simulink:masks:SFunctionBuilder_MD
      mask init: same as gate_V31_2016a/Gate/HardwareInputWrapper/HIL/S-Function Builder1
      settings: CopyFcn=set_param(gcbh,'LinkStatus','none')  PreSaveFcn=try, set_param(gcb,'FunctionName','BluetoothLib'), end  OpenFcn=sfunctionwizard(gcbh)
      mask Display: plot(val(:,1),val(:,2)),disp(sys), port_label('output',1,'BtCharRecieved')
  S-Function Builder1 : S-Function  Commented=on  InitFcn=try, set_param(gcb,'FunctionName','printingBlock'), end  FunctionName=printingBlock  SFunctionModules=printingBlock_wrapper  SFunctionDeploymentMode=off  EnableBusSupport=off  mask(S-Function Builder){}
      mask help: Simulink:masks:SFunctionBuilder_MD
      mask init: same as gate_V31_2016a/Gate/HardwareInputWrapper/HIL/S-Function Builder1
      settings: CopyFcn=set_param(gcbh,'LinkStatus','none')  PreSaveFcn=try, set_param(gcb,'FunctionName','printingBlock'), end  OpenFcn=sfunctionwizard(gcbh)
      mask Display: plot(val(:,1),val(:,2)),disp(sys), port_label('input',1,'u0'), port_label('output',1,'y0')
  Unit Delay : UnitDelay  Commented=on  SampleTime=-1
  bluetoothCharPressed : Outport
  wires:
  S-Function Builder/1 -> S-Function Builder1/1, Unit Delay/1, bluetoothCharPressed/1
  Unit Delay/1 -> ?
